simulink library "inp"  (Simulink 8.2)
settings: Solver=ode45  SolverMode=SingleTasking  StartTime=0.0  StopTime=10.0  RelTol=1e-3
undefined symbols (not defined in the file or in its folder): C_TICK_TIME_S, ENUM_FAULTSTATE_FAIL, ENUM_FAULTSTATE_INDETERMINATE, ENUM_FAULTSTATE_PASS, ENUM_KEYSWITCH_ON, FALSE, KINP_ABS1CANCommuOk_flg_ovrdflg, KINP_ABS1CANCommuOk_flg_ovrdval, KINP_ABS1CANPeriodTime_s, KINP_ABS1CANRolCntOk_flg_ovrdflg, KINP_ABS1CANRolCntOk_flg_ovrdval, KINP_ABS2CANChkSumOk_flg_ovrdflg, KINP_ABS2CANChkSumOk_flg_ovrdval, KINP_ABS2CANCommuOk_flg_ovrdflg, KINP_ABS2CANCommuOk_flg_ovrdval, KINP_ABS2CANPeriodTime_s, KINP_ABS2CANRolCntOk_flg_ovrdflg, KINP_ABS2CANRolCntOk_flg_ovrdval, KINP_ABS3CANCommuOk_flg_ovrdflg, KINP_ABS3CANCommuOk_flg_ovrdval, KINP_ABS4CANCommuOk_flg_ovrdflg, KINP_ABS4CANCommuOk_flg_ovrdval, KINP_ABSActive_flg_defval, KINP_ABSActive_flg_invalid, KINP_ABSActive_flg_ovrdflg, KINP_ABSActive_flg_ovrdval, KINP_ABSActive_flg_setvalid, KINP_ABSActive_flg_srvcflg, KINP_ABSActive_flg_srvcval, KINP_ABSCANAlive_flg_ovrdflg, KINP_ABSCANAlive_flg_ovrdval, KINP_ABSCANCommuOk_flg_ovrdflg, KINP_ABSCANCommuOk_flg_ovrdval, KINP_ABSCANValidOk_flg_ovrdflg, KINP_ABSCANValidOk_flg_ovrdval, KINP_ABSFault_flg_defval, KINP_ABSFault_flg_invalid, KINP_ABSFault_flg_ovrdflg, KINP_ABSFault_flg_ovrdval, KINP_ABSFault_flg_setvalid, KINP_ABSFault_flg_srvcflg, KINP_ABSFault_flg_srvcval, KINP_AC1CANCommuOk_flg_ovrdflg, KINP_AC1CANCommuOk_flg_ovrdval, KINP_ACCANAlive_flg_ovrdflg, KINP_ACCANAlive_flg_ovrdval, KINP_ACCANCommuOk_flg_ovrdflg, KINP_ACCANCommuOk_flg_ovrdval, KINP_ACCANValidOk_flg_ovrdflg, KINP_ACCANValidOk_flg_ovrdval, KINP_ACCCancelH_flg_defval, KINP_ACCCancelH_flg_invalid, KINP_ACCCancelH_flg_ovrdflg, KINP_ACCCancelH_flg_ovrdval, KINP_ACCCancelH_flg_setvalid, KINP_ACCCancelH_flg_srvcflg, KINP_ACCCancelH_flg_srvcval, KINP_AccelPedalPosn_pct_defval, KINP_AccelPedalPosn_pct_gain, KINP_AccelPedalPosn_pct_invalid ... (+1866)
libraries: inp x192, hcu_lib x34, simulink x33
(part 7 of 21)

== system inp/INP/BCM_signals/KeySwitchStatus ==
  signal_in : Inport
  msg_valid : Inport  Port=2
  Active_fault_flg : Inport  Port=3
  can_signal_process_wio_validity : inp/can_signal_process_wio_validity
  defval : Constant  Value=KINP_KeySwitchStatus_enum_defval
  invalid : Constant  Value=KINP_KeySwitchStatus_enum_invalid
  ovrdflg : Constant  Value=KINP_KeySwitchStatus_enum_ovrdflg
  ovrdval : Constant  Value=KINP_KeySwitchStatus_enum_ovrdval
  setvalid : Constant  Value=KINP_KeySwitchStatus_enum_setvalid
  srvcflg : Constant  Value=KINP_KeySwitchStatus_enum_srvcflg
  srvcval : Constant  Value=KINP_KeySwitchStatus_enum_srvcval
  signal_out : Outport
  signal_valid_out : Outport  Port=2
  wires:
  can_signal_process_wio_validity/1 -> signal_out/1
  can_signal_process_wio_validity/2 -> signal_valid_out/1
  signal_in/1 -> can_signal_process_wio_validity/1
  msg_valid/1 -> can_signal_process_wio_validity/2
  defval/1 -> can_signal_process_wio_validity/4
  invalid/1 -> can_signal_process_wio_validity/5
  setvalid/1 -> can_signal_process_wio_validity/6
  ovrdflg/1 -> can_signal_process_wio_validity/7
  ovrdval/1 -> can_signal_process_wio_validity/8
  srvcflg/1 -> can_signal_process_wio_validity/9
  srvcval/1 -> can_signal_process_wio_validity/10
  Active_fault_flg/1 -> can_signal_process_wio_validity/3

== system inp/INP/BCM_signals/LRDoorOpen ==
  signal_in : Inport
  msg_valid : Inport  Port=2
  Active_fault_flg : Inport  Port=3
  can_signal_process_wio_validity : inp/can_signal_process_wio_validity
  defval : Constant  Value=KINP_LRDoorOpen_flg_defval
  invalid : Constant  Value=KINP_LRDoorOpen_flg_invalid
  ovrdflg : Constant  Value=KINP_LRDoorOpen_flg_ovrdflg
  ovrdval : Constant  Value=KINP_LRDoorOpen_flg_ovrdval
  setvalid : Constant  Value=KINP_LRDoorOpen_flg_setvalid
  srvcflg : Constant  Value=KINP_LRDoorOpen_flg_srvcflg
  srvcval : Constant  Value=KINP_LRDoorOpen_flg_srvcval
  signal_out : Outport
  signal_valid_out : Outport  Port=2
  wires:
  Active_fault_flg/1 -> can_signal_process_wio_validity/3
  srvcval/1 -> can_signal_process_wio_validity/10
  srvcflg/1 -> can_signal_process_wio_validity/9
  ovrdval/1 -> can_signal_process_wio_validity/8
  ovrdflg/1 -> can_signal_process_wio_validity/7
  setvalid/1 -> can_signal_process_wio_validity/6
  invalid/1 -> can_signal_process_wio_validity/5
  defval/1 -> can_signal_process_wio_validity/4
  msg_valid/1 -> can_signal_process_wio_validity/2
  signal_in/1 -> can_signal_process_wio_validity/1
  can_signal_process_wio_validity/2 -> signal_valid_out/1
  can_signal_process_wio_validity/1 -> signal_out/1

== system inp/INP/BCM_signals/PassengerDoorOpen ==
  signal_in : Inport
  msg_valid : Inport  Port=2
  Active_fault_flg : Inport  Port=3
  can_signal_process_wio_validity : inp/can_signal_process_wio_validity
  defval : Constant  Value=KINP_PassengerDoorOpen_flg_defval
  invalid : Constant  Value=KINP_PassengerDoorOpen_flg_invalid
  ovrdflg : Constant  Value=KINP_PassengerDoorOpen_flg_ovrdflg
  ovrdval : Constant  Value=KINP_PassengerDoorOpen_flg_ovrdval
  setvalid : Constant  Value=KINP_PassengerDoorOpen_flg_setvalid
  srvcflg : Constant  Value=KINP_PassengerDoorOpen_flg_srvcflg
  srvcval : Constant  Value=KINP_PassengerDoorOpen_flg_srvcval
  signal_out : Outport
  signal_valid_out : Outport  Port=2
  wires:
  can_signal_process_wio_validity/1 -> signal_out/1
  can_signal_process_wio_validity/2 -> signal_valid_out/1
  signal_in/1 -> can_signal_process_wio_validity/1
  msg_valid/1 -> can_signal_process_wio_validity/2
  defval/1 -> can_signal_process_wio_validity/4
  invalid/1 -> can_signal_process_wio_validity/5
  setvalid/1 -> can_signal_process_wio_validity/6
  ovrdflg/1 -> can_signal_process_wio_validity/7
  ovrdval/1 -> can_signal_process_wio_validity/8
  srvcflg/1 -> can_signal_process_wio_validity/9
  srvcval/1 -> can_signal_process_wio_validity/10
  Active_fault_flg/1 -> can_signal_process_wio_validity/3

== system inp/INP/BCM_signals/RRDoorOpen ==
  signal_in : Inport
  msg_valid : Inport  Port=2
  Active_fault_flg : Inport  Port=3
  can_signal_process_wio_validity : inp/can_signal_process_wio_validity
  defval : Constant  Value=KINP_RRDoorOpen_flg_defval
  invalid : Constant  Value=KINP_RRDoorOpen_flg_invalid
  ovrdflg : Constant  Value=KINP_RRDoorOpen_flg_ovrdflg
  ovrdval : Constant  Value=KINP_RRDoorOpen_flg_ovrdval
  setvalid : Constant  Value=KINP_RRDoorOpen_flg_setvalid
  srvcflg : Constant  Value=KINP_RRDoorOpen_flg_srvcflg
  srvcval : Constant  Value=KINP_RRDoorOpen_flg_srvcval
  signal_out : Outport
  signal_valid_out : Outport  Port=2
  wires:
  can_signal_process_wio_validity/1 -> signal_out/1
  can_signal_process_wio_validity/2 -> signal_valid_out/1
  signal_in/1 -> can_signal_process_wio_validity/1
  msg_valid/1 -> can_signal_process_wio_validity/2
  defval/1 -> can_signal_process_wio_validity/4
  invalid/1 -> can_signal_process_wio_validity/5
  setvalid/1 -> can_signal_process_wio_validity/6
  ovrdflg/1 -> can_signal_process_wio_validity/7
  ovrdval/1 -> can_signal_process_wio_validity/8
  srvcflg/1 -> can_signal_process_wio_validity/9
  srvcval/1 -> can_signal_process_wio_validity/10
  Active_fault_flg/1 -> can_signal_process_wio_validity/3

== system inp/INP/BCM_signals/TailGateOpen ==
  signal_in : Inport
  msg_valid : Inport  Port=2
  Active_fault_flg : Inport  Port=3
  can_signal_process_wio_validity : inp/can_signal_process_wio_validity
  defval : Constant  Value=KINP_TailGateOpen_flg_defval
  invalid : Constant  Value=KINP_TailGateOpen_flg_invalid
  ovrdflg : Constant  Value=KINP_TailGateOpen_flg_ovrdflg
  ovrdval : Constant  Value=KINP_TailGateOpen_flg_ovrdval
  setvalid : Constant  Value=KINP_TailGateOpen_flg_setvalid
  srvcflg : Constant  Value=KINP_TailGateOpen_flg_srvcflg
  srvcval : Constant  Value=KINP_TailGateOpen_flg_srvcval
  signal_out : Outport
  signal_valid_out : Outport  Port=2
  wires:
  Active_fault_flg/1 -> can_signal_process_wio_validity/3
  srvcval/1 -> can_signal_process_wio_validity/10
  srvcflg/1 -> can_signal_process_wio_validity/9
  ovrdval/1 -> can_signal_process_wio_validity/8
  ovrdflg/1 -> can_signal_process_wio_validity/7
  setvalid/1 -> can_signal_process_wio_validity/6
  invalid/1 -> can_signal_process_wio_validity/5
  defval/1 -> can_signal_process_wio_validity/4
  msg_valid/1 -> can_signal_process_wio_validity/2
  signal_in/1 -> can_signal_process_wio_validity/1
  can_signal_process_wio_validity/2 -> signal_valid_out/1
  can_signal_process_wio_validity/1 -> signal_out/1

== system inp/INP/BCM_signals/TurnSignalSwLeft ==
  signal_in : Inport
  msg_valid : Inport  Port=2
  Active_fault_flg : Inport  Port=3
  can_signal_process_wio_validity : inp/can_signal_process_wio_validity
  defval : Constant  Value=KINP_TurnSignalSwL_flg_defval
  invalid : Constant  Value=KINP_TurnSignalSwL_flg_invalid
  ovrdflg : Constant  Value=KINP_TurnSignalSwL_flg_ovrdflg
  ovrdval : Constant  Value=KINP_TurnSignalSwL_flg_ovrdval
  setvalid : Constant  Value=KINP_TurnSignalSwL_flg_setvalid
  srvcflg : Constant  Value=KINP_TurnSignalSwL_flg_srvcflg
  srvcval : Constant  Value=KINP_TurnSignalSwL_flg_srvcval
  signal_out : Outport
  signal_valid_out : Outport  Port=2
  wires:
  Active_fault_flg/1 -> can_signal_process_wio_validity/3
  srvcval/1 -> can_signal_process_wio_validity/10
  srvcflg/1 -> can_signal_process_wio_validity/9
  ovrdval/1 -> can_signal_process_wio_validity/8
  ovrdflg/1 -> can_signal_process_wio_validity/7
  setvalid/1 -> can_signal_process_wio_validity/6
  invalid/1 -> can_signal_process_wio_validity/5
  defval/1 -> can_signal_process_wio_validity/4
  msg_valid/1 -> can_signal_process_wio_validity/2
  signal_in/1 -> can_signal_process_wio_validity/1
  can_signal_process_wio_validity/2 -> signal_valid_out/1
  can_signal_process_wio_validity/1 -> signal_out/1

== system inp/INP/BCM_signals/TurnSignalSwRight ==
  signal_in : Inport
  msg_valid : Inport  Port=2
  Active_fault_flg : Inport  Port=3
  can_signal_process_wio_validity : inp/can_signal_process_wio_validity
  defval : Constant  Value=KINP_TurnSignalSwR_flg_defval
  invalid : Constant  Value=KINP_TurnSignalSwR_flg_invalid
  ovrdflg : Constant  Value=KINP_TurnSignalSwR_flg_ovrdflg
  ovrdval : Constant  Value=KINP_TurnSignalSwR_flg_ovrdval
  setvalid : Constant  Value=KINP_TurnSignalSwR_flg_setvalid
  srvcflg : Constant  Value=KINP_TurnSignalSwR_flg_srvcflg
  srvcval : Constant  Value=KINP_TurnSignalSwR_flg_srvcval
  signal_out : Outport
  signal_valid_out : Outport  Port=2
  wires:
  can_signal_process_wio_validity/1 -> signal_out/1
  can_signal_process_wio_validity/2 -> signal_valid_out/1
  signal_in/1 -> can_signal_process_wio_validity/1
  msg_valid/1 -> can_signal_process_wio_validity/2
  defval/1 -> can_signal_process_wio_validity/4
  invalid/1 -> can_signal_process_wio_validity/5
  setvalid/1 -> can_signal_process_wio_validity/6
  ovrdflg/1 -> can_signal_process_wio_validity/7
  ovrdval/1 -> can_signal_process_wio_validity/8
  srvcflg/1 -> can_signal_process_wio_validity/9
  srvcval/1 -> can_signal_process_wio_validity/10
  Active_fault_flg/1 -> can_signal_process_wio_validity/3

== system inp/INP/BCM_signals/VehCrashed ==
  signal_in : Inport
  signal_valid_in : Inport  Port=2
  msg_valid : Inport  Port=3
  Active_fault_flg : Inport  Port=4
  can_signal_process_with_validity : inp/can_signal_process_with_validity
  defval : Constant  Value=KINP_VehCrashed_flg_defval
  invalid : Constant  Value=KINP_VehCrashed_flg_invalid
  ovrdflg : Constant  Value=KINP_VehCrashed_flg_ovrdflg
  ovrdval : Constant  Value=KINP_VehCrashed_flg_ovrdval
  setvalid : Constant  Value=KINP_VehCrashed_flg_setvalid
  srvcflg : Constant  Value=KINP_VehCrashed_flg_srvcflg
  srvcval : Constant  Value=KINP_VehCrashed_flg_srvcval
  signal_out : Outport
  signal_valid_out : Outport  Port=2
  raw_signal_valid_out : Outport  Port=3
  wires:
  srvcval/1 -> can_signal_process_with_validity/12
  srvcflg/1 -> can_signal_process_with_validity/11
  ovrdval/1 -> can_signal_process_with_validity/10
  ovrdflg/1 -> can_signal_process_with_validity/9
  setvalid/1 -> can_signal_process_with_validity/8
  invalid/1 -> can_signal_process_with_validity/7
  defval/1 -> can_signal_process_with_validity/6
  can_signal_process_with_validity/3 -> raw_signal_valid_out/1
  can_signal_process_with_validity/1 -> signal_out/1
  can_signal_process_with_validity/2 -> signal_valid_out/1
  signal_in/1 -> can_signal_process_with_validity/1
  signal_valid_in/1 -> can_signal_process_with_validity/2
  msg_valid/1 -> can_signal_process_with_validity/5, can_signal_process_with_validity/3
  Active_fault_flg/1 -> can_signal_process_with_validity/4

== system inp/INP/CAN_Message_Valid_Proc/ABS1Updated ==
  signal_in : Inport
  diagnosis_switch : Inport  Port=2
  Active_fault_flg : Inport  Port=3
  can_valid_process : inp/can_valid_process
  defval : Constant  Value=KINP_CANMsgUpdatedABS1_flg_defval
  invalid : Constant  Value=KINP_CANMsgUpdatedABS1_flg_invalid
  ovrdflg : Constant  Value=KINP_CANMsgUpdatedABS1_flg_ovrdflg
  ovrdval : Constant  Value=KINP_CANMsgUpdatedABS1_flg_ovrdval
  setvalid : Constant  Value=KINP_CANMsgUpdatedABS1_flg_setvalid
  srvcflg : Constant  Value=KINP_CANMsgUpdatedABS1_flg_srvcflg
  srvcval : Constant  Value=KINP_CANMsgUpdatedABS1_flg_srvcval
  tempval : Constant  Value=KINP_CANMsgUpdatedABS1_flg_tempval
  signal_out : Outport
  signal_valid_out : Outport  Port=2
  wires:
  srvcval/1 -> can_valid_process/11
  srvcflg/1 -> can_valid_process/10
  ovrdval/1 -> can_valid_process/9
  ovrdflg/1 -> can_valid_process/8
  setvalid/1 -> can_valid_process/7
  invalid/1 -> can_valid_process/6
  tempval/1 -> can_valid_process/5
  defval/1 -> can_valid_process/4
  diagnosis_switch/1 -> can_valid_process/3
  can_valid_process/1 -> signal_out/1
  can_valid_process/2 -> signal_valid_out/1
  signal_in/1 -> can_valid_process/1
  Active_fault_flg/1 -> can_valid_process/2

== system inp/INP/CAN_Message_Valid_Proc/ABS1Valid ==
  signal_in : Inport
  Active_fault_flg : Inport  Port=2
  TRUE : Constant  Value=TRUE
  can_valid_process : inp/can_valid_process
  defval : Constant  Value=KINP_CANMsgValidABS1_flg_defval
  invalid : Constant  Value=KINP_CANMsgValidABS1_flg_invalid
  ovrdflg : Constant  Value=KINP_CANMsgValidABS1_flg_ovrdflg
  ovrdval : Constant  Value=KINP_CANMsgValidABS1_flg_ovrdval
  setvalid : Constant  Value=KINP_CANMsgValidABS1_flg_setvalid
  srvcflg : Constant  Value=KINP_CANMsgValidABS1_flg_srvcflg
  srvcval : Constant  Value=KINP_CANMsgValidABS1_flg_srvcval
  tempval : Constant  Value=KINP_CANMsgValidABS1_flg_tempval
  signal_out : Outport
  signal_valid_out : Outport  Port=2
  wires:
  srvcval/1 -> can_valid_process/11
  srvcflg/1 -> can_valid_process/10
  ovrdval/1 -> can_valid_process/9
  ovrdflg/1 -> can_valid_process/8
  setvalid/1 -> can_valid_process/7
  invalid/1 -> can_valid_process/6
  tempval/1 -> can_valid_process/5
  defval/1 -> can_valid_process/4
  TRUE/1 -> can_valid_process/3
  Active_fault_flg/1 -> can_valid_process/2
  signal_in/1 -> can_valid_process/1
  can_valid_process/2 -> signal_valid_out/1
  can_valid_process/1 -> signal_out/1

== system inp/INP/CAN_Message_Valid_Proc/ABS2Checked ==
  signal_in : Inport
  diagnosis_switch : Inport  Port=2
  Active_fault_flg : Inport  Port=3
  can_valid_process : inp/can_valid_process
  defval : Constant  Value=KINP_CANMsgCheckedABS2_flg_defval
  invalid : Constant  Value=KINP_CANMsgCheckedABS2_flg_invalid
  ovrdflg : Constant  Value=KINP_CANMsgCheckedABS2_flg_ovrdflg
  ovrdval : Constant  Value=KINP_CANMsgCheckedABS2_flg_ovrdval
  setvalid : Constant  Value=KINP_CANMsgCheckedABS2_flg_setvalid
  srvcflg : Constant  Value=KINP_CANMsgCheckedABS2_flg_srvcflg
  srvcval : Constant  Value=KINP_CANMsgCheckedABS2_flg_srvcval
  tempval : Constant  Value=KINP_CANMsgCheckedABS2_flg_tempval
  signal_out : Outport
  signal_valid_out : Outport  Port=2
  wires:
  srvcval/1 -> can_valid_process/11
  srvcflg/1 -> can_valid_process/10
  ovrdval/1 -> can_valid_process/9
  ovrdflg/1 -> can_valid_process/8
  setvalid/1 -> can_valid_process/7
  invalid/1 -> can_valid_process/6
  tempval/1 -> can_valid_process/5
  defval/1 -> can_valid_process/4
  diagnosis_switch/1 -> can_valid_process/3
  can_valid_process/1 -> signal_out/1
  can_valid_process/2 -> signal_valid_out/1
  signal_in/1 -> can_valid_process/1
  Active_fault_flg/1 -> can_valid_process/2

== system inp/INP/CAN_Message_Valid_Proc/ABS2Updated ==
  signal_in : Inport
  diagnosis_switch : Inport  Port=2
  Active_fault_flg : Inport  Port=3
  can_valid_process : inp/can_valid_process
  defval : Constant  Value=KINP_CANMsgUpdatedABS2_flg_defval
  invalid : Constant  Value=KINP_CANMsgUpdatedABS2_flg_invalid
  ovrdflg : Constant  Value=KINP_CANMsgUpdatedABS2_flg_ovrdflg
  ovrdval : Constant  Value=KINP_CANMsgUpdatedABS2_flg_ovrdval
  setvalid : Constant  Value=KINP_CANMsgUpdatedABS2_flg_setvalid
  srvcflg : Constant  Value=KINP_CANMsgUpdatedABS2_flg_srvcflg
  srvcval : Constant  Value=KINP_CANMsgUpdatedABS2_flg_srvcval
  tempval : Constant  Value=KINP_CANMsgUpdatedABS2_flg_tempval
  signal_out : Outport
  signal_valid_out : Outport  Port=2
  wires:
  Active_fault_flg/1 -> can_valid_process/2
  signal_in/1 -> can_valid_process/1
  can_valid_process/2 -> signal_valid_out/1
  can_valid_process/1 -> signal_out/1
  diagnosis_switch/1 -> can_valid_process/3
  defval/1 -> can_valid_process/4
  tempval/1 -> can_valid_process/5
  invalid/1 -> can_valid_process/6
  setvalid/1 -> can_valid_process/7
  ovrdflg/1 -> can_valid_process/8
  ovrdval/1 -> can_valid_process/9
  srvcflg/1 -> can_valid_process/10
  srvcval/1 -> can_valid_process/11

== system inp/INP/CAN_Message_Valid_Proc/ABS2Valid ==
  signal_in : Inport
  Active_fault_flg : Inport  Port=2
  TRUE : Constant  Value=TRUE
  can_valid_process : inp/can_valid_process
  defval : Constant  Value=KINP_CANMsgValidABS2_flg_defval
  invalid : Constant  Value=KINP_CANMsgValidABS2_flg_invalid
  ovrdflg : Constant  Value=KINP_CANMsgValidABS2_flg_ovrdflg
  ovrdval : Constant  Value=KINP_CANMsgValidABS2_flg_ovrdval
  setvalid : Constant  Value=KINP_CANMsgValidABS2_flg_setvalid
  srvcflg : Constant  Value=KINP_CANMsgValidABS2_flg_srvcflg
  srvcval : Constant  Value=KINP_CANMsgValidABS2_flg_srvcval
  tempval : Constant  Value=KINP_CANMsgValidABS2_flg_tempval
  signal_out : Outport
  signal_valid_out : Outport  Port=2
  wires:
  can_valid_process/1 -> signal_out/1
  can_valid_process/2 -> signal_valid_out/1
  signal_in/1 -> can_valid_process/1
  Active_fault_flg/1 -> can_valid_process/2
  TRUE/1 -> can_valid_process/3
  defval/1 -> can_valid_process/4
  tempval/1 -> can_valid_process/5
  invalid/1 -> can_valid_process/6
  setvalid/1 -> can_valid_process/7
  ovrdflg/1 -> can_valid_process/8
  ovrdval/1 -> can_valid_process/9
  srvcflg/1 -> can_valid_process/10
  srvcval/1 -> can_valid_process/11

== system inp/INP/CAN_Message_Valid_Proc/ABS3Valid ==
  signal_in : Inport
  Active_fault_flg : Inport  Port=2
  TRUE : Constant  Value=TRUE
  can_valid_process : inp/can_valid_process
  defval : Constant  Value=KINP_CANMsgValidABS3_flg_defval
  invalid : Constant  Value=KINP_CANMsgValidABS3_flg_invalid
  ovrdflg : Constant  Value=KINP_CANMsgValidABS3_flg_ovrdflg
  ovrdval : Constant  Value=KINP_CANMsgValidABS3_flg_ovrdval
  setvalid : Constant  Value=KINP_CANMsgValidABS3_flg_setvalid
  srvcflg : Constant  Value=KINP_CANMsgValidABS3_flg_srvcflg
  srvcval : Constant  Value=KINP_CANMsgValidABS3_flg_srvcval
  tempval : Constant  Value=KINP_CANMsgValidABS3_flg_tempval
  signal_out : Outport
  signal_valid_out : Outport  Port=2
  wires:
  srvcval/1 -> can_valid_process/11
  srvcflg/1 -> can_valid_process/10
  ovrdval/1 -> can_valid_process/9
  ovrdflg/1 -> can_valid_process/8
  setvalid/1 -> can_valid_process/7
  invalid/1 -> can_valid_process/6
  tempval/1 -> can_valid_process/5
  defval/1 -> can_valid_process/4
  TRUE/1 -> can_valid_process/3
  Active_fault_flg/1 -> can_valid_process/2
  signal_in/1 -> can_valid_process/1
  can_valid_process/2 -> signal_valid_out/1
  can_valid_process/1 -> signal_out/1

== system inp/INP/CAN_Message_Valid_Proc/ABS4Valid ==
  signal_in : Inport
  Active_fault_flg : Inport  Port=2
  TRUE : Constant  Value=TRUE
  can_valid_process : inp/can_valid_process
  defval : Constant  Value=KINP_CANMsgValidABS4_flg_defval
  invalid : Constant  Value=KINP_CANMsgValidABS4_flg_invalid
  ovrdflg : Constant  Value=KINP_CANMsgValidABS4_flg_ovrdflg
  ovrdval : Constant  Value=KINP_CANMsgValidABS4_flg_ovrdval
  setvalid : Constant  Value=KINP_CANMsgValidABS4_flg_setvalid
  srvcflg : Constant  Value=KINP_CANMsgValidABS4_flg_srvcflg
  srvcval : Constant  Value=KINP_CANMsgValidABS4_flg_srvcval
  tempval : Constant  Value=KINP_CANMsgValidABS4_flg_tempval
  signal_out : Outport
  signal_valid_out : Outport  Port=2
  wires:
  Active_fault_flg/1 -> can_valid_process/2
  signal_in/1 -> can_valid_process/1
  can_valid_process/2 -> signal_valid_out/1
  can_valid_process/1 -> signal_out/1
  TRUE/1 -> can_valid_process/3
  defval/1 -> can_valid_process/4
  tempval/1 -> can_valid_process/5
  invalid/1 -> can_valid_process/6
  setvalid/1 -> can_valid_process/7
  ovrdflg/1 -> can_valid_process/8
  ovrdval/1 -> can_valid_process/9
  srvcflg/1 -> can_valid_process/10
  srvcval/1 -> can_valid_process/11

== system inp/INP/CAN_Message_Valid_Proc/AC1Valid ==
  signal_in : Inport
  Active_fault_flg : Inport  Port=2
  TRUE : Constant  Value=TRUE
  can_valid_process : inp/can_valid_process
  defval : Constant  Value=KINP_CANMsgValidAC1_flg_defval
  invalid : Constant  Value=KINP_CANMsgValidAC1_flg_invalid
  ovrdflg : Constant  Value=KINP_CANMsgValidAC1_flg_ovrdflg
  ovrdval : Constant  Value=KINP_CANMsgValidAC1_flg_ovrdval
  setvalid : Constant  Value=KINP_CANMsgValidAC1_flg_setvalid
  srvcflg : Constant  Value=KINP_CANMsgValidAC1_flg_srvcflg
  srvcval : Constant  Value=KINP_CANMsgValidAC1_flg_srvcval
  tempval : Constant  Value=KINP_CANMsgValidAC1_flg_tempval
  signal_out : Outport
  signal_valid_out : Outport  Port=2
  wires:
  srvcval/1 -> can_valid_process/11
  srvcflg/1 -> can_valid_process/10
  ovrdval/1 -> can_valid_process/9
  ovrdflg/1 -> can_valid_process/8
  setvalid/1 -> can_valid_process/7
  invalid/1 -> can_valid_process/6
  tempval/1 -> can_valid_process/5
  defval/1 -> can_valid_process/4
  TRUE/1 -> can_valid_process/3
  Active_fault_flg/1 -> can_valid_process/2
  signal_in/1 -> can_valid_process/1
  can_valid_process/2 -> signal_valid_out/1
  can_valid_process/1 -> signal_out/1

== system inp/INP/CAN_Message_Valid_Proc/BCM1Valid ==
  signal_in : Inport
  Active_fault_flg : Inport  Port=2
  TRUE : Constant  Value=TRUE
  can_valid_process : inp/can_valid_process
  defval : Constant  Value=KINP_CANMsgValidBCM1_flg_defval
  invalid : Constant  Value=KINP_CANMsgValidBCM1_flg_invalid
  ovrdflg : Constant  Value=KINP_CANMsgValidBCM1_flg_ovrdflg
  ovrdval : Constant  Value=KINP_CANMsgValidBCM1_flg_ovrdval
  setvalid : Constant  Value=KINP_CANMsgValidBCM1_flg_setvalid
  srvcflg : Constant  Value=KINP_CANMsgValidBCM1_flg_srvcflg
  srvcval : Constant  Value=KINP_CANMsgValidBCM1_flg_srvcval
  tempval : Constant  Value=KINP_CANMsgValidBCM1_flg_tempval
  signal_out : Outport
  signal_valid_out : Outport  Port=2
  wires:
  can_valid_process/1 -> signal_out/1
  can_valid_process/2 -> signal_valid_out/1
  signal_in/1 -> can_valid_process/1
  Active_fault_flg/1 -> can_valid_process/2
  TRUE/1 -> can_valid_process/3
  defval/1 -> can_valid_process/4
  tempval/1 -> can_valid_process/5
  invalid/1 -> can_valid_process/6
  setvalid/1 -> can_valid_process/7
  ovrdflg/1 -> can_valid_process/8
  ovrdval/1 -> can_valid_process/9
  srvcflg/1 -> can_valid_process/10
  srvcval/1 -> can_valid_process/11

== system inp/INP/CAN_Message_Valid_Proc/BCM2Valid ==
  signal_in : Inport
  Active_fault_flg : Inport  Port=2
  TRUE : Constant  Value=TRUE
  can_valid_process : inp/can_valid_process
  defval : Constant  Value=KINP_CANMsgValidBCM2_flg_defval
  invalid : Constant  Value=KINP_CANMsgValidBCM2_flg_invalid
  ovrdflg : Constant  Value=KINP_CANMsgValidBCM2_flg_ovrdflg
  ovrdval : Constant  Value=KINP_CANMsgValidBCM2_flg_ovrdval
  setvalid : Constant  Value=KINP_CANMsgValidBCM2_flg_setvalid
  srvcflg : Constant  Value=KINP_CANMsgValidBCM2_flg_srvcflg
  srvcval : Constant  Value=KINP_CANMsgValidBCM2_flg_srvcval
  tempval : Constant  Value=KINP_CANMsgValidBCM2_flg_tempval
  signal_out : Outport
  signal_valid_out : Outport  Port=2
  wires:
  srvcval/1 -> can_valid_process/11
  srvcflg/1 -> can_valid_process/10
  ovrdval/1 -> can_valid_process/9
  ovrdflg/1 -> can_valid_process/8
  setvalid/1 -> can_valid_process/7
  invalid/1 -> can_valid_process/6
  tempval/1 -> can_valid_process/5
  defval/1 -> can_valid_process/4
  TRUE/1 -> can_valid_process/3
  Active_fault_flg/1 -> can_valid_process/2
  signal_in/1 -> can_valid_process/1
  can_valid_process/2 -> signal_valid_out/1
  can_valid_process/1 -> signal_out/1

== system inp/INP/CAN_Message_Valid_Proc/CSW1Valid ==
  signal_in : Inport
  Active_fault_flg : Inport  Port=2
  TRUE : Constant  Value=TRUE
  can_valid_process : inp/can_valid_process
  defval : Constant  Value=KINP_CANMsgValidCSW1_flg_defval
  invalid : Constant  Value=KINP_CANMsgValidCSW1_flg_invalid
  ovrdflg : Constant  Value=KINP_CANMsgValidCSW1_flg_ovrdflg
  ovrdval : Constant  Value=KINP_CANMsgValidCSW1_flg_ovrdval
  setvalid : Constant  Value=KINP_CANMsgValidCSW1_flg_setvalid
  srvcflg : Constant  Value=KINP_CANMsgValidCSW1_flg_srvcflg
  srvcval : Constant  Value=KINP_CANMsgValidCSW1_flg_srvcval
  tempval : Constant  Value=KINP_CANMsgValidCSW1_flg_tempval
  signal_out : Outport
  signal_valid_out : Outport  Port=2
  wires:
  can_valid_process/1 -> signal_out/1
  can_valid_process/2 -> signal_valid_out/1
  signal_in/1 -> can_valid_process/1
  Active_fault_flg/1 -> can_valid_process/2
  TRUE/1 -> can_valid_process/3
  defval/1 -> can_valid_process/4
  tempval/1 -> can_valid_process/5
  invalid/1 -> can_valid_process/6
  setvalid/1 -> can_valid_process/7
  ovrdflg/1 -> can_valid_process/8
  ovrdval/1 -> can_valid_process/9
  srvcflg/1 -> can_valid_process/10
  srvcval/1 -> can_valid_process/11

== system inp/INP/CAN_Message_Valid_Proc/ECM1Valid ==
  signal_in : Inport
  Active_fault_flg : Inport  Port=2
  TRUE : Constant  Value=TRUE
  can_valid_process : inp/can_valid_process
  defval : Constant  Value=KINP_CANMsgValidECM1_flg_defval
  invalid : Constant  Value=KINP_CANMsgValidECM1_flg_invalid
  ovrdflg : Constant  Value=KINP_CANMsgValidECM1_flg_ovrdflg
  ovrdval : Constant  Value=KINP_CANMsgValidECM1_flg_ovrdval
  setvalid : Constant  Value=KINP_CANMsgValidECM1_flg_setvalid
  srvcflg : Constant  Value=KINP_CANMsgValidECM1_flg_srvcflg
  srvcval : Constant  Value=KINP_CANMsgValidECM1_flg_srvcval
  tempval : Constant  Value=KINP_CANMsgValidECM1_flg_tempval
  signal_out : Outport
  signal_valid_out : Outport  Port=2
  wires:
  srvcval/1 -> can_valid_process/11
  srvcflg/1 -> can_valid_process/10
  ovrdval/1 -> can_valid_process/9
  ovrdflg/1 -> can_valid_process/8
  setvalid/1 -> can_valid_process/7
  invalid/1 -> can_valid_process/6
  tempval/1 -> can_valid_process/5
  defval/1 -> can_valid_process/4
  TRUE/1 -> can_valid_process/3
  can_valid_process/1 -> signal_out/1
  can_valid_process/2 -> signal_valid_out/1
  signal_in/1 -> can_valid_process/1
  Active_fault_flg/1 -> can_valid_process/2

== system inp/INP/CAN_Message_Valid_Proc/ECM2Valid ==
  signal_in : Inport
  Active_fault_flg : Inport  Port=2
  TRUE : Constant  Value=TRUE
  can_valid_process : inp/can_valid_process
  defval : Constant  Value=KINP_CANMsgValidECM2_flg_defval
  invalid : Constant  Value=KINP_CANMsgValidECM2_flg_invalid
  ovrdflg : Constant  Value=KINP_CANMsgValidECM2_flg_ovrdflg
  ovrdval : Constant  Value=KINP_CANMsgValidECM2_flg_ovrdval
  setvalid : Constant  Value=KINP_CANMsgValidECM2_flg_setvalid
  srvcflg : Constant  Value=KINP_CANMsgValidECM2_flg_srvcflg
  srvcval : Constant  Value=KINP_CANMsgValidECM2_flg_srvcval
  tempval : Constant  Value=KINP_CANMsgValidECM2_flg_tempval
  signal_out : Outport
  signal_valid_out : Outport  Port=2
  wires:
  Active_fault_flg/1 -> can_valid_process/2
  signal_in/1 -> can_valid_process/1
  can_valid_process/2 -> signal_valid_out/1
  can_valid_process/1 -> signal_out/1
  TRUE/1 -> can_valid_process/3
  defval/1 -> can_valid_process/4
  tempval/1 -> can_valid_process/5
  invalid/1 -> can_valid_process/6
  setvalid/1 -> can_valid_process/7
  ovrdflg/1 -> can_valid_process/8
  ovrdval/1 -> can_valid_process/9
  srvcflg/1 -> can_valid_process/10
  srvcval/1 -> can_valid_process/11

== system inp/INP/CAN_Message_Valid_Proc/ECM3Valid ==
  signal_in : Inport
  Active_fault_flg : Inport  Port=2
  TRUE : Constant  Value=TRUE
  can_valid_process : inp/can_valid_process
  defval : Constant  Value=KINP_CANMsgValidECM3_flg_defval
  invalid : Constant  Value=KINP_CANMsgValidECM3_flg_invalid
  ovrdflg : Constant  Value=KINP_CANMsgValidECM3_flg_ovrdflg
  ovrdval : Constant  Value=KINP_CANMsgValidECM3_flg_ovrdval
  setvalid : Constant  Value=KINP_CANMsgValidECM3_flg_setvalid
  srvcflg : Constant  Value=KINP_CANMsgValidECM3_flg_srvcflg
  srvcval : Constant  Value=KINP_CANMsgValidECM3_flg_srvcval
  tempval : Constant  Value=KINP_CANMsgValidECM3_flg_tempval
  signal_out : Outport
  signal_valid_out : Outport  Port=2
  wires:
  Active_fault_flg/1 -> can_valid_process/2
  signal_in/1 -> can_valid_process/1
  can_valid_process/2 -> signal_valid_out/1
  can_valid_process/1 -> signal_out/1
  TRUE/1 -> can_valid_process/3
  defval/1 -> can_valid_process/4
  tempval/1 -> can_valid_process/5
  invalid/1 -> can_valid_process/6
  setvalid/1 -> can_valid_process/7
  ovrdflg/1 -> can_valid_process/8
  ovrdval/1 -> can_valid_process/9
  srvcflg/1 -> can_valid_process/10
  srvcval/1 -> can_valid_process/11

== system inp/INP/CAN_Message_Valid_Proc/ECM9Updated ==
  signal_in : Inport
  diagnosis_switch : Inport  Port=2
  Active_fault_flg : Inport  Port=3
  can_valid_process : inp/can_valid_process
  defval : Constant  Value=KINP_CANMsgUpdatedECM9_flg_defval
  invalid : Constant  Value=KINP_CANMsgUpdatedECM9_flg_invalid
  ovrdflg : Constant  Value=KINP_CANMsgUpdatedECM9_flg_ovrdflg
  ovrdval : Constant  Value=KINP_CANMsgUpdatedECM9_flg_ovrdval
  setvalid : Constant  Value=KINP_CANMsgUpdatedECM9_flg_setvalid
  srvcflg : Constant  Value=KINP_CANMsgUpdatedECM9_flg_srvcflg
  srvcval : Constant  Value=KINP_CANMsgUpdatedECM9_flg_srvcval
  tempval : Constant  Value=KINP_CANMsgUpdatedECM9_flg_tempval
  signal_out : Outport
  signal_valid_out : Outport  Port=2
  wires:
  Active_fault_flg/1 -> can_valid_process/2
  signal_in/1 -> can_valid_process/1
  can_valid_process/2 -> signal_valid_out/1
  can_valid_process/1 -> signal_out/1
  diagnosis_switch/1 -> can_valid_process/3
  defval/1 -> can_valid_process/4
  tempval/1 -> can_valid_process/5
  invalid/1 -> can_valid_process/6
  setvalid/1 -> can_valid_process/7
  ovrdflg/1 -> can_valid_process/8
  ovrdval/1 -> can_valid_process/9
  srvcflg/1 -> can_valid_process/10
  srvcval/1 -> can_valid_process/11

== system inp/INP/CAN_Message_Valid_Proc/ECM9Valid ==
  signal_in : Inport
  Active_fault_flg : Inport  Port=2
  TRUE : Constant  Value=TRUE
  can_valid_process : inp/can_valid_process
  defval : Constant  Value=KINP_CANMsgValidECM9_flg_defval
  invalid : Constant  Value=KINP_CANMsgValidECM9_flg_invalid
  ovrdflg : Constant  Value=KINP_CANMsgValidECM9_flg_ovrdflg
  ovrdval : Constant  Value=KINP_CANMsgValidECM9_flg_ovrdval
  setvalid : Constant  Value=KINP_CANMsgValidECM9_flg_setvalid
  srvcflg : Constant  Value=KINP_CANMsgValidECM9_flg_srvcflg
  srvcval : Constant  Value=KINP_CANMsgValidECM9_flg_srvcval
  tempval : Constant  Value=KINP_CANMsgValidECM9_flg_tempval
  signal_out : Outport
  signal_valid_out : Outport  Port=2
  wires:
  can_valid_process/1 -> signal_out/1
  can_valid_process/2 -> signal_valid_out/1
  signal_in/1 -> can_valid_process/1
  Active_fault_flg/1 -> can_valid_process/2
  TRUE/1 -> can_valid_process/3
  defval/1 -> can_valid_process/4
  tempval/1 -> can_valid_process/5
  invalid/1 -> can_valid_process/6
  setvalid/1 -> can_valid_process/7
  ovrdflg/1 -> can_valid_process/8
  ovrdval/1 -> can_valid_process/9
  srvcflg/1 -> can_valid_process/10
  srvcval/1 -> can_valid_process/11

== system inp/INP/CAN_Message_Valid_Proc/EPAS1Valid ==
  signal_in : Inport
  Active_fault_flg : Inport  Port=2
  TRUE : Constant  Value=TRUE
  can_valid_process : inp/can_valid_process
  defval : Constant  Value=KINP_CANMsgValidEPAS1_flg_defval
  invalid : Constant  Value=KINP_CANMsgValidEPAS1_flg_invalid
  ovrdflg : Constant  Value=KINP_CANMsgValidEPAS1_flg_ovrdflg
  ovrdval : Constant  Value=KINP_CANMsgValidEPAS1_flg_ovrdval
  setvalid : Constant  Value=KINP_CANMsgValidEPAS1_flg_setvalid
  srvcflg : Constant  Value=KINP_CANMsgValidEPAS1_flg_srvcflg
  srvcval : Constant  Value=KINP_CANMsgValidEPAS1_flg_srvcval
  tempval : Constant  Value=KINP_CANMsgValidEPAS1_flg_tempval
  signal_out : Outport
  signal_valid_out : Outport  Port=2
  wires:
  can_valid_process/1 -> signal_out/1
  can_valid_process/2 -> signal_valid_out/1
  signal_in/1 -> can_valid_process/1
  Active_fault_flg/1 -> can_valid_process/2
  TRUE/1 -> can_valid_process/3
  defval/1 -> can_valid_process/4
  tempval/1 -> can_valid_process/5
  invalid/1 -> can_valid_process/6
  setvalid/1 -> can_valid_process/7
  ovrdflg/1 -> can_valid_process/8
  ovrdval/1 -> can_valid_process/9
  srvcflg/1 -> can_valid_process/10
  srvcval/1 -> can_valid_process/11

== system inp/INP/CAN_Message_Valid_Proc/EPAS2Checked ==
  signal_in : Inport
  diagnosis_switch : Inport  Port=2
  Active_fault_flg : Inport  Port=3
  can_valid_process : inp/can_valid_process
  defval : Constant  Value=KINP_CANMsgCheckedEPAS2_flg_defval
  invalid : Constant  Value=KINP_CANMsgCheckedEPAS2_flg_invalid
  ovrdflg : Constant  Value=KINP_CANMsgCheckedEPAS2_flg_ovrdflg
  ovrdval : Constant  Value=KINP_CANMsgCheckedEPAS2_flg_ovrdval
  setvalid : Constant  Value=KINP_CANMsgCheckedEPAS2_flg_setvalid
  srvcflg : Constant  Value=KINP_CANMsgCheckedEPAS2_flg_srvcflg
  srvcval : Constant  Value=KINP_CANMsgCheckedEPAS2_flg_srvcval
  tempval : Constant  Value=KINP_CANMsgCheckedEPAS2_flg_tempval
  signal_out : Outport
  signal_valid_out : Outport  Port=2
  wires:
  can_valid_process/1 -> signal_out/1
  can_valid_process/2 -> signal_valid_out/1
  signal_in/1 -> can_valid_process/1
  Active_fault_flg/1 -> can_valid_process/2
  diagnosis_switch/1 -> can_valid_process/3
  defval/1 -> can_valid_process/4
  tempval/1 -> can_valid_process/5
  invalid/1 -> can_valid_process/6
  setvalid/1 -> can_valid_process/7
  ovrdflg/1 -> can_valid_process/8
  ovrdval/1 -> can_valid_process/9
  srvcflg/1 -> can_valid_process/10
  srvcval/1 -> can_valid_process/11

== system inp/INP/CAN_Message_Valid_Proc/EPAS2Updated ==
  signal_in : Inport
  diagnosis_switch : Inport  Port=2
  Active_fault_flg : Inport  Port=3
  can_valid_process : inp/can_valid_process
  defval : Constant  Value=KINP_CANMsgUpdatedEPAS2_flg_defval
  invalid : Constant  Value=KINP_CANMsgUpdatedEPAS2_flg_invalid
  ovrdflg : Constant  Value=KINP_CANMsgUpdatedEPAS2_flg_ovrdflg
  ovrdval : Constant  Value=KINP_CANMsgUpdatedEPAS2_flg_ovrdval
  setvalid : Constant  Value=KINP_CANMsgUpdatedEPAS2_flg_setvalid
  srvcflg : Constant  Value=KINP_CANMsgUpdatedEPAS2_flg_srvcflg
  srvcval : Constant  Value=KINP_CANMsgUpdatedEPAS2_flg_srvcval
  tempval : Constant  Value=KINP_CANMsgUpdatedEPAS2_flg_tempval
  signal_out : Outport
  signal_valid_out : Outport  Port=2
  wires:
  srvcval/1 -> can_valid_process/11
  srvcflg/1 -> can_valid_process/10
  ovrdval/1 -> can_valid_process/9
  ovrdflg/1 -> can_valid_process/8
  setvalid/1 -> can_valid_process/7
  invalid/1 -> can_valid_process/6
  tempval/1 -> can_valid_process/5
  defval/1 -> can_valid_process/4
  diagnosis_switch/1 -> can_valid_process/3
  Active_fault_flg/1 -> can_valid_process/2
  signal_in/1 -> can_valid_process/1
  can_valid_process/2 -> signal_valid_out/1
  can_valid_process/1 -> signal_out/1

== system inp/INP/CAN_Message_Valid_Proc/EPAS2Valid ==
  signal_in : Inport
  Active_fault_flg : Inport  Port=2
  TRUE : Constant  Value=TRUE
  can_valid_process : inp/can_valid_process
  defval : Constant  Value=KINP_CANMsgValidEPAS2_flg_defval
  invalid : Constant  Value=KINP_CANMsgValidEPAS2_flg_invalid
  ovrdflg : Constant  Value=KINP_CANMsgValidEPAS2_flg_ovrdflg
  ovrdval : Constant  Value=KINP_CANMsgValidEPAS2_flg_ovrdval
  setvalid : Constant  Value=KINP_CANMsgValidEPAS2_flg_setvalid
  srvcflg : Constant  Value=KINP_CANMsgValidEPAS2_flg_srvcflg
  srvcval : Constant  Value=KINP_CANMsgValidEPAS2_flg_srvcval
  tempval : Constant  Value=KINP_CANMsgValidEPAS2_flg_tempval
  signal_out : Outport
  signal_valid_out : Outport  Port=2
  wires:
  srvcval/1 -> can_valid_process/11
  srvcflg/1 -> can_valid_process/10
  ovrdval/1 -> can_valid_process/9
  ovrdflg/1 -> can_valid_process/8
  setvalid/1 -> can_valid_process/7
  invalid/1 -> can_valid_process/6
  tempval/1 -> can_valid_process/5
  defval/1 -> can_valid_process/4
  TRUE/1 -> can_valid_process/3
  Active_fault_flg/1 -> can_valid_process/2
  signal_in/1 -> can_valid_process/1
  can_valid_process/2 -> signal_valid_out/1
  can_valid_process/1 -> signal_out/1

== system inp/INP/CAN_Message_Valid_Proc/EPAS3Checked ==
  signal_in : Inport
  diagnosis_switch : Inport  Port=2
  Active_fault_flg : Inport  Port=3
  can_valid_process : inp/can_valid_process
  defval : Constant  Value=KINP_CANMsgCheckedEPAS3_flg_defval
  invalid : Constant  Value=KINP_CANMsgCheckedEPAS3_flg_invalid
  ovrdflg : Constant  Value=KINP_CANMsgCheckedEPAS3_flg_ovrdflg
  ovrdval : Constant  Value=KINP_CANMsgCheckedEPAS3_flg_ovrdval
  setvalid : Constant  Value=KINP_CANMsgCheckedEPAS3_flg_setvalid
  srvcflg : Constant  Value=KINP_CANMsgCheckedEPAS3_flg_srvcflg
  srvcval : Constant  Value=KINP_CANMsgCheckedEPAS3_flg_srvcval
  tempval : Constant  Value=KINP_CANMsgCheckedEPAS3_flg_tempval
  signal_out : Outport
  signal_valid_out : Outport  Port=2
  wires:
  srvcval/1 -> can_valid_process/11
  srvcflg/1 -> can_valid_process/10
  ovrdval/1 -> can_valid_process/9
  ovrdflg/1 -> can_valid_process/8
  setvalid/1 -> can_valid_process/7
  invalid/1 -> can_valid_process/6
  tempval/1 -> can_valid_process/5
  defval/1 -> can_valid_process/4
  diagnosis_switch/1 -> can_valid_process/3
  Active_fault_flg/1 -> can_valid_process/2
  signal_in/1 -> can_valid_process/1
  can_valid_process/2 -> signal_valid_out/1
  can_valid_process/1 -> signal_out/1

== system inp/INP/CAN_Message_Valid_Proc/EPAS3Updated ==
  signal_in : Inport
  diagnosis_switch : Inport  Port=2
  Active_fault_flg : Inport  Port=3
  can_valid_process : inp/can_valid_process
  defval : Constant  Value=KINP_CANMsgUpdatedEPAS3_flg_defval
  invalid : Constant  Value=KINP_CANMsgUpdatedEPAS3_flg_invalid
  ovrdflg : Constant  Value=KINP_CANMsgUpdatedEPAS3_flg_ovrdflg
  ovrdval : Constant  Value=KINP_CANMsgUpdatedEPAS3_flg_ovrdval
  setvalid : Constant  Value=KINP_CANMsgUpdatedEPAS3_flg_setvalid
  srvcflg : Constant  Value=KINP_CANMsgUpdatedEPAS3_flg_srvcflg
  srvcval : Constant  Value=KINP_CANMsgUpdatedEPAS3_flg_srvcval
  tempval : Constant  Value=KINP_CANMsgUpdatedEPAS3_flg_tempval
  signal_out : Outport
  signal_valid_out : Outport  Port=2
  wires:
  can_valid_process/1 -> signal_out/1
  can_valid_process/2 -> signal_valid_out/1
  signal_in/1 -> can_valid_process/1
  Active_fault_flg/1 -> can_valid_process/2
  diagnosis_switch/1 -> can_valid_process/3
  defval/1 -> can_valid_process/4
  tempval/1 -> can_valid_process/5
  invalid/1 -> can_valid_process/6
  setvalid/1 -> can_valid_process/7
  ovrdflg/1 -> can_valid_process/8
  ovrdval/1 -> can_valid_process/9
  srvcflg/1 -> can_valid_process/10
  srvcval/1 -> can_valid_process/11

== system inp/INP/CAN_Message_Valid_Proc/EPAS3Valid ==
  signal_in : Inport
  Active_fault_flg : Inport  Port=2
  TRUE : Constant  Value=TRUE
  can_valid_process : inp/can_valid_process
  defval : Constant  Value=KINP_CANMsgValidEPAS3_flg_defval
  invalid : Constant  Value=KINP_CANMsgValidEPAS3_flg_invalid
  ovrdflg : Constant  Value=KINP_CANMsgValidEPAS3_flg_ovrdflg
  ovrdval : Constant  Value=KINP_CANMsgValidEPAS3_flg_ovrdval
  setvalid : Constant  Value=KINP_CANMsgValidEPAS3_flg_setvalid
  srvcflg : Constant  Value=KINP_CANMsgValidEPAS3_flg_srvcflg
  srvcval : Constant  Value=KINP_CANMsgValidEPAS3_flg_srvcval
  tempval : Constant  Value=KINP_CANMsgValidEPAS3_flg_tempval
  signal_out : Outport
  signal_valid_out : Outport  Port=2
  wires:
  can_valid_process/1 -> signal_out/1
  can_valid_process/2 -> signal_valid_out/1
  signal_in/1 -> can_valid_process/1
  Active_fault_flg/1 -> can_valid_process/2
  TRUE/1 -> can_valid_process/3
  defval/1 -> can_valid_process/4
  tempval/1 -> can_valid_process/5
  invalid/1 -> can_valid_process/6
  setvalid/1 -> can_valid_process/7
  ovrdflg/1 -> can_valid_process/8
  ovrdval/1 -> can_valid_process/9
  srvcflg/1 -> can_valid_process/10
  srvcval/1 -> can_valid_process/11

== system inp/INP/CAN_Message_Valid_Proc/EPB1Valid ==
  signal_in : Inport
  Active_fault_flg : Inport  Port=2
  TRUE : Constant  Value=TRUE
  can_valid_process : inp/can_valid_process
  defval : Constant  Value=KINP_CANMsgValidEPB1_flg_defval
  invalid : Constant  Value=KINP_CANMsgValidEPB1_flg_invalid
  ovrdflg : Constant  Value=KINP_CANMsgValidEPB1_flg_ovrdflg
  ovrdval : Constant  Value=KINP_CANMsgValidEPB1_flg_ovrdval
  setvalid : Constant  Value=KINP_CANMsgValidEPB1_flg_setvalid
  srvcflg : Constant  Value=KINP_CANMsgValidEPB1_flg_srvcflg
  srvcval : Constant  Value=KINP_CANMsgValidEPB1_flg_srvcval
  tempval : Constant  Value=KINP_CANMsgValidEPB1_flg_tempval
  signal_out : Outport
  signal_valid_out : Outport  Port=2
  wires:
  can_valid_process/1 -> signal_out/1
  can_valid_process/2 -> signal_valid_out/1
  signal_in/1 -> can_valid_process/1
  Active_fault_flg/1 -> can_valid_process/2
  TRUE/1 -> can_valid_process/3
  defval/1 -> can_valid_process/4
  tempval/1 -> can_valid_process/5
  invalid/1 -> can_valid_process/6
  setvalid/1 -> can_valid_process/7
  ovrdflg/1 -> can_valid_process/8
  ovrdval/1 -> can_valid_process/9
  srvcflg/1 -> can_valid_process/10
  srvcval/1 -> can_valid_process/11

== system inp/INP/CAN_Message_Valid_Proc/EPMCheckReceived ==
  BHAL_outputs : Inport
  Bus Creator : BusCreator  Inputs=12
  Bus Creator1 : BusCreator  Inputs=12
  Bus Selector5 : BusSelector  OutputSignals=VHAL_CamLeftLaneReceiveValid_flg,VHAL_CamRightLaneReceiveValid_flg,VHAL_CamObs1ReceiveValid_flg,VHAL_CamObs2ReceiveValid_flg,VHAL_CamObs3ReceiveValid_flg,VHAL_CamObs4ReceiveValid_flg,VHAL_CamObs5ReceiveValid_flg,VHAL_CamObs6ReceiveValid_flg,VHAL_CamObs7ReceiveValid_flg,VHAL_CamObs8ReceiveValid_flg,VHAL_CamObs9ReceiveValid_flg,VHAL_CamObs10ReceiveValid_flg
  Constant{1..24} (24 alike) : Constant  Value=TRUE
  Terminator{1..12} (12 alike) : Terminator
  VINP_CamCANReceiveValid_flg : Outport
  VINP_CamCANReceiveOk_flg : Outport  Port=2
  wires:
  Bus Creator1/1 -> VINP_CamCANReceiveOk_flg/1
  Bus Creator/1 -> VINP_CamCANReceiveValid_flg/1
  BHAL_outputs/1 -> Bus Selector5/1
  Constant11/1 -> [VINP_CamLeftLaneReceiveOk_flg]  "VINP_CamLeftLaneReceiveOk_flg"
  Constant10/1 -> [VINP_CamLeftLaneReceiveValid_flg]  "VINP_CamLeftLaneReceiveValid_flg"
  Bus Selector5/1 -> Terminator5/1  "<VHAL_CamLeftLaneReceiveValid_flg>"
  Constant13/1 -> [VINP_CamRightLaneReceiveOk_flg]  "VINP_CamRightLaneReceiveOk_flg"
  Constant12/1 -> [VINP_CamRightLaneReceiveValid_flg]  "VINP_CamRightLaneReceiveValid_flg"
  Bus Selector5/2 -> Terminator6/1  "<VHAL_CamRightLaneReceiveValid_flg>"
  Constant15/1 -> [VINP_CamObs1ReceiveOk_flg]  "VINP_CamObs1ReceiveOk_flg"
  Constant14/1 -> [VINP_CamObs1ReceiveValid_flg]  "VINP_CamObs1ReceiveValid_flg"
  Bus Selector5/3 -> Terminator7/1  "<VHAL_CamObs1ReceiveValid_flg>"
  Constant17/1 -> [VINP_CamObs2ReceiveOk_flg]  "VINP_CamObs2ReceiveOk_flg"
  Constant16/1 -> [VINP_CamObs2ReceiveValid_flg]  "VINP_CamObs2ReceiveValid_flg"
  Bus Selector5/4 -> Terminator8/1  "<VHAL_CamObs2ReceiveValid_flg>"
  Constant19/1 -> [VINP_CamObs3ReceiveOk_flg]  "VINP_CamObs3ReceiveOk_flg"
  Constant18/1 -> [VINP_CamObs3ReceiveValid_flg]  "VINP_CamObs3ReceiveValid_flg"
  Bus Selector5/5 -> Terminator9/1  "<VHAL_CamObs3ReceiveValid_flg>"
  Constant21/1 -> [VINP_CamObs4ReceiveOk_flg]  "VINP_CamObs4ReceiveOk_flg"
  Constant20/1 -> [VINP_CamObs4ReceiveValid_flg]  "VINP_CamObs4ReceiveValid_flg"
  Bus Selector5/6 -> Terminator10/1  "<VHAL_CamObs4ReceiveValid_flg>"
  Constant23/1 -> [VINP_CamObs5ReceiveOk_flg]  "VINP_CamObs5ReceiveOk_flg"
  Constant22/1 -> [VINP_CamObs5ReceiveValid_flg]  "VINP_CamObs5ReceiveValid_flg"
  Bus Selector5/7 -> Terminator11/1  "<VHAL_CamObs5ReceiveValid_flg>"
  Constant2/1 -> [VINP_CamObs6ReceiveOk_flg]  "VINP_CamObs6ReceiveOk_flg"
  Constant1/1 -> [VINP_CamObs6ReceiveValid_flg]  "VINP_CamObs6ReceiveValid_flg"
  Bus Selector5/8 -> Terminator1/1  "<VHAL_CamObs6ReceiveValid_flg>"
  Constant4/1 -> [VINP_CamObs7ReceiveOk_flg]  "VINP_CamObs7ReceiveOk_flg"
  Constant3/1 -> [VINP_CamObs7ReceiveValid_flg]  "VINP_CamObs7ReceiveValid_flg"
  Bus Selector5/9 -> Terminator2/1  "<VHAL_CamObs7ReceiveValid_flg>"
  Constant6/1 -> [VINP_CamObs8ReceiveOk_flg]  "VINP_CamObs8ReceiveOk_flg"
  Constant5/1 -> [VINP_CamObs8ReceiveValid_flg]  "VINP_CamObs8ReceiveValid_flg"
  Bus Selector5/10 -> Terminator3/1  "<VHAL_CamObs8ReceiveValid_flg>"
  Constant8/1 -> [VINP_CamObs9ReceiveOk_flg]  "VINP_CamObs9ReceiveOk_flg"
  Constant7/1 -> [VINP_CamObs9ReceiveValid_flg]  "VINP_CamObs9ReceiveValid_flg"
  Bus Selector5/11 -> Terminator4/1  "<VHAL_CamObs9ReceiveValid_flg>"
  Constant24/1 -> [VINP_CamObs10ReceiveOk_flg]  "VINP_CamObs10ReceiveOk_flg"
  Constant9/1 -> [VINP_CamObs10ReceiveValid_flg]  "VINP_CamObs10ReceiveValid_flg"
  Bus Selector5/12 -> Terminator12/1  "<VHAL_CamObs10ReceiveValid_flg>"
  [VINP_CamRightLaneReceiveValid_flg] -> Bus Creator/2
  [VINP_CamLeftLaneReceiveValid_flg] -> Bus Creator/1
  [VINP_CamObs1ReceiveValid_flg] -> Bus Creator/3
  [VINP_CamObs3ReceiveValid_flg] -> Bus Creator/5
  [VINP_CamObs2ReceiveValid_flg] -> Bus Creator/4
  [VINP_CamObs6ReceiveValid_flg] -> Bus Creator/8
  [VINP_CamObs10ReceiveValid_flg] -> Bus Creator/12
  [VINP_CamObs5ReceiveValid_flg] -> Bus Creator/7
  [VINP_CamObs9ReceiveValid_flg] -> Bus Creator/11
  [VINP_CamObs4ReceiveValid_flg] -> Bus Creator/6
  [VINP_CamObs7ReceiveValid_flg] -> Bus Creator/9
  [VINP_CamObs8ReceiveValid_flg] -> Bus Creator/10
  [VINP_CamLeftLaneReceiveOk_flg] -> Bus Creator1/1
  [VINP_CamRightLaneReceiveOk_flg] -> Bus Creator1/2
  [VINP_CamObs1ReceiveOk_flg] -> Bus Creator1/3
  [VINP_CamObs9ReceiveOk_flg] -> Bus Creator1/11
  [VINP_CamObs6ReceiveOk_flg] -> Bus Creator1/8
  [VINP_CamObs4ReceiveOk_flg] -> Bus Creator1/6
  [VINP_CamObs2ReceiveOk_flg] -> Bus Creator1/4
  [VINP_CamObs3ReceiveOk_flg] -> Bus Creator1/5
  [VINP_CamObs10ReceiveOk_flg] -> Bus Creator1/12
  [VINP_CamObs8ReceiveOk_flg] -> Bus Creator1/10
  [VINP_CamObs5ReceiveOk_flg] -> Bus Creator1/7
  [VINP_CamObs7ReceiveOk_flg] -> Bus Creator1/9
